simulink model "x_vacc_test"  (Simulink 8.0)
settings: Solver=ode45  StartTime=0.0  StopTime=20*(4096/8)*40  RelTol=1e-3
libraries: xbsIndex_r4 x531
(part 2 of 4)

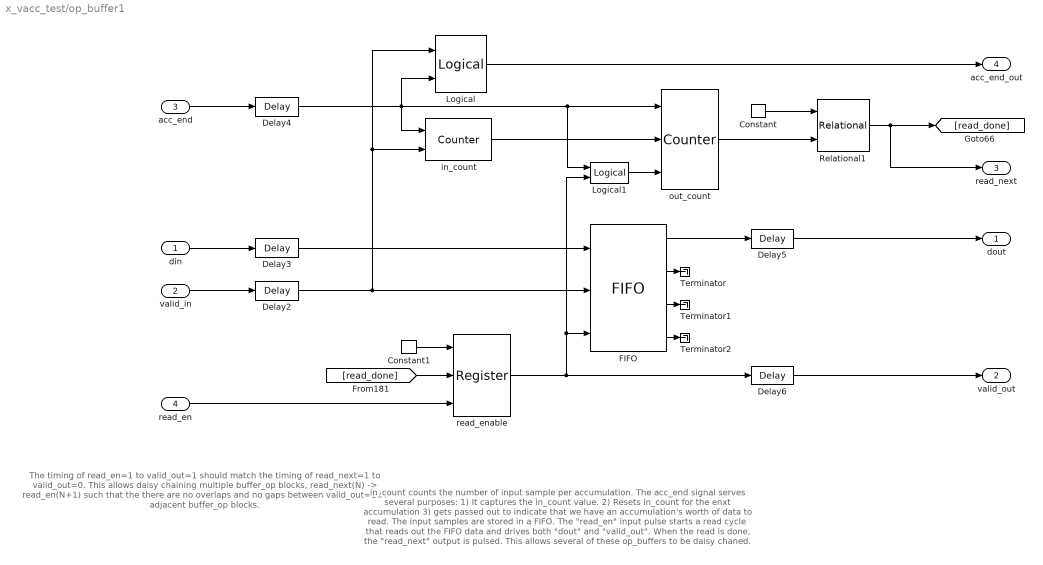
== system x_vacc_test/op_buffer1 ==
  note: The timing of read_en=1 to valid_out=1 should
      | match the timing of read_next=1 to valid_out=0.
      |
      | This allows daisy chaining multiple buffer_op blocks,
      | read_next(N) -> read_en(N+1) such that the there are
      | no overlaps and no gaps between valid_out=1 of
      | adjacent buffer_op blocks.
  note: in_count counts the number of input sample per accumulation.
      |
      | The acc_end signal serves several purposes:
      |
      | 1) It captures the in_count value.
      | 2) Resets in_count for the enxt accumulation
      | 3) gets passed out to indicate that we have an accumulation's
      | worth of data  to read.
      |
      | The input samples are stored in a FIFO.  The "read_en" input pulse
      | starts a read cycle that reads out the FIFO data and drives both
      | "dout" and "valid_out".  When the read is done, the "read_next"
      | output is pulsed.  This allows several of these op_buffers to be
      | daisy chaned.
  din : Inport
  valid_in : Inport  Port=2
  acc_end : Inport  Port=3
  read_en : Inport  Port=4
  Constant : xbsIndex_r4/Constant  const=1  gui_display_data_type=Fixed-point  arith_type=Unsigned  n_bits=1  bin_pt=0  preci_type=Single  exp_width=8  frac_width=24  explicit_period=on  period=1  equ=P=C  opselect=C  inp2=PCIN>>17  opr=+  inp1=P  carry=CIN  dbl_ovrd=off  block_type=constant
  Constant1 : xbsIndex_r4/Constant  const=1  gui_display_data_type=Boolean  arith_type=Boolean  n_bits=16  bin_pt=14  preci_type=Single  exp_width=8  frac_width=24  explicit_period=on  period=1  equ=P=C  opselect=C  inp2=PCIN>>17  opr=+  inp1=P  carry=CIN  dbl_ovrd=off  block_type=constant
  Delay{2..6} (5 alike) : xbsIndex_r4/Delay  rst=off  infoeditControl=Selection of Reset will increase slice count due to use of real FFs and instead of SRLs  en=off  latency=1  dbl_ovrd=off  reg_retiming=on  block_type=delay
      settings: block_version=11.4
  FIFO : xbsIndex_r4/FIFO  mem_type=Block RAM  performance_options=First_Word_Fall_Through  infoedit1=Available only for V4, V5, V6, V7, K7, A7 and Zynq devices.  embedded_registers=on  depth=8K  percent_nbits=1  rst=off  en=off  use_dcount=off  use_percent_full_port=on  use_almost_empty=off  almost_empty_thresh=2  use_almost_full=off  almost_full_thresh=14  dbl_ovrd=off  explicit_period=off  block_type=fifo
  Logical : xbsIndex_r4/Logical  logical_function=AND  inputs=2  en=off  latency=1  precision=Full  arith_type=Unsigned  n_bits=16  bin_pt=0  align_bp=on  dbl_ovrd=off  block_type=logical
  Logical1 : xbsIndex_r4/Logical  logical_function=OR  inputs=2  en=off  latency=0  precision=Full  arith_type=Unsigned  n_bits=16  bin_pt=0  align_bp=on  dbl_ovrd=off  block_type=logical
  Relational1 : xbsIndex_r4/Relational  mode=a=b  en=off  latency=1  dbl_ovrd=off  block_type=relational
  Terminator : Terminator
  Terminator1 : Terminator
  Terminator2 : Terminator
  in_count : xbsIndex_r4/Counter  cnt_type=Free Running  cnt_to=n_buffer * n_x_op * (2 ^ (freq_chan - n_x_eng)) - 1  operation=Up  start_count=0  cnt_by_val=1  arith_type=Unsigned  n_bits=nextpow2((n_x_op/2) * (2 ^ (freq_chan - n_x_eng)))  bin_pt=0  load_pin=off  rst=on  en=on  explicit_period=off  period=1  dbl_ovrd=off  use_behavioral_HDL=off  implementation=Fabric  block_type=counter
      settings: block_version=11.4
  out_count : xbsIndex_r4/Counter  cnt_type=Free Running  cnt_to=n_buffer * n_x_op * (2 ^ (freq_chan - n_x_eng)) - 1  operation=Down  start_count=0  cnt_by_val=1  arith_type=Unsigned  n_bits=nextpow2((n_x_op/2) * (2 ^ (freq_chan - n_x_eng)))  bin_pt=0  load_pin=on  rst=off  en=on  explicit_period=off  period=1  dbl_ovrd=off  use_behavioral_HDL=off  implementation=Fabric  block_type=counter
      settings: block_version=11.4
  read_enable : xbsIndex_r4/Register  init=0  rst=on  en=on  dbl_ovrd=off  block_type=register
  dout : Outport
  valid_out : Outport  Port=2
  read_next : Outport  Port=3
  acc_end_out : Outport  Port=4
  wires:
  acc_end/1 -> Delay4/1
  in_count/1 -> out_count/2
  valid_in/1 -> Delay2/1
  read_enable/1 -> Delay6/1, Logical1/2, FIFO/3
  out_count/1 -> Relational1/2
  din/1 -> Delay3/1
  Relational1/1 -> read_next/1, [read_done]
  [read_done] -> read_enable/2
  Delay6/1 -> valid_out/1
  FIFO/1 -> Delay5/1
  Logical/1 -> acc_end_out/1
  read_en/1 -> read_enable/3
  Delay2/1 -> FIFO/2, Logical/1, in_count/2
  Delay3/1 -> FIFO/1
  Delay4/1 -> Logical/2, out_count/1, Logical1/1, in_count/1
  FIFO/2 -> Terminator/1
  FIFO/3 -> Terminator1/1
  FIFO/4 -> Terminator2/1
  Constant/1 -> Relational1/1
  Constant1/1 -> read_enable/1
  Delay5/1 -> dout/1
  Logical1/1 -> out_count/3

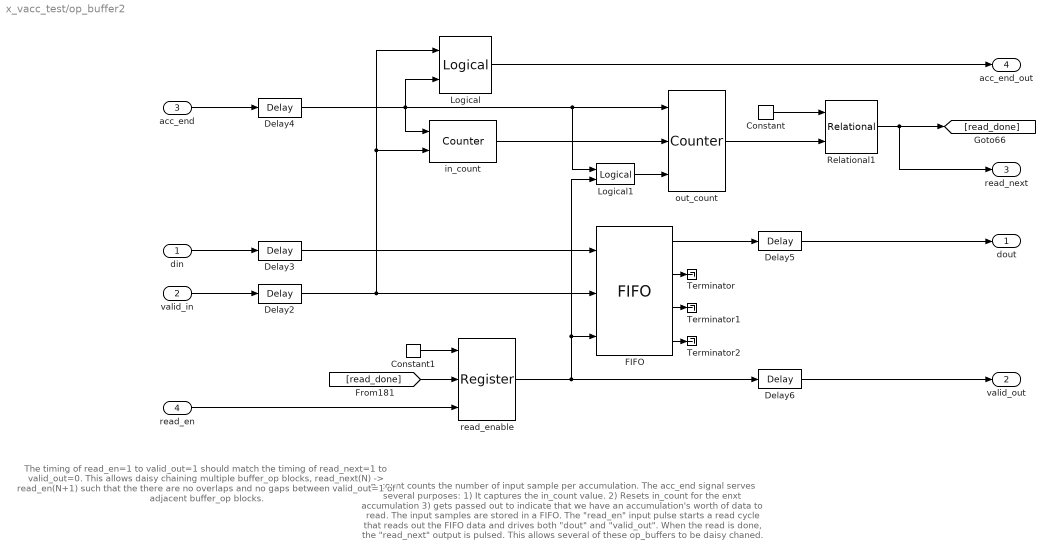
== system x_vacc_test/op_buffer2 ==
  note: in_count counts the number of input sample per accumulation.
      |
      | The acc_end signal serves several purposes:
      |
      | 1) It captures the in_count value.
      | 2) Resets in_count for the enxt accumulation
      | 3) gets passed out to indicate that we have an accumulation's
      | worth of data  to read.
      |
      | The input samples are stored in a FIFO.  The "read_en" input pulse
      | starts a read cycle that reads out the FIFO data and drives both
      | "dout" and "valid_out".  When the read is done, the "read_next"
      | output is pulsed.  This allows several of these op_buffers to be
      | daisy chaned.
  note: The timing of read_en=1 to valid_out=1 should
      | match the timing of read_next=1 to valid_out=0.
      |
      | This allows daisy chaining multiple buffer_op blocks,
      | read_next(N) -> read_en(N+1) such that the there are
      | no overlaps and no gaps between valid_out=1 of
      | adjacent buffer_op blocks.
  din : Inport
  valid_in : Inport  Port=2
  acc_end : Inport  Port=3
  read_en : Inport  Port=4
  Constant : xbsIndex_r4/Constant  const=1  gui_display_data_type=Fixed-point  arith_type=Unsigned  n_bits=1  bin_pt=0  preci_type=Single  exp_width=8  frac_width=24  explicit_period=on  period=1  equ=P=C  opselect=C  inp2=PCIN>>17  opr=+  inp1=P  carry=CIN  dbl_ovrd=off  block_type=constant
  Constant1 : xbsIndex_r4/Constant  const=1  gui_display_data_type=Boolean  arith_type=Boolean  n_bits=16  bin_pt=14  preci_type=Single  exp_width=8  frac_width=24  explicit_period=on  period=1  equ=P=C  opselect=C  inp2=PCIN>>17  opr=+  inp1=P  carry=CIN  dbl_ovrd=off  block_type=constant
  Delay{2..6} (5 alike) : xbsIndex_r4/Delay  rst=off  infoeditControl=Selection of Reset will increase slice count due to use of real FFs and instead of SRLs  en=off  latency=1  dbl_ovrd=off  reg_retiming=on  block_type=delay
      settings: block_version=11.4
  FIFO : xbsIndex_r4/FIFO  mem_type=Block RAM  performance_options=First_Word_Fall_Through  infoedit1=Available only for V4, V5, V6, V7, K7, A7 and Zynq devices.  embedded_registers=on  depth=8K  percent_nbits=1  rst=off  en=off  use_dcount=off  use_percent_full_port=on  use_almost_empty=off  almost_empty_thresh=2  use_almost_full=off  almost_full_thresh=14  dbl_ovrd=off  explicit_period=off  block_type=fifo
  Logical : xbsIndex_r4/Logical  logical_function=AND  inputs=2  en=off  latency=1  precision=Full  arith_type=Unsigned  n_bits=16  bin_pt=0  align_bp=on  dbl_ovrd=off  block_type=logical
  Logical1 : xbsIndex_r4/Logical  logical_function=OR  inputs=2  en=off  latency=0  precision=Full  arith_type=Unsigned  n_bits=16  bin_pt=0  align_bp=on  dbl_ovrd=off  block_type=logical
  Relational1 : xbsIndex_r4/Relational  mode=a=b  en=off  latency=1  dbl_ovrd=off  block_type=relational
  Terminator : Terminator
  Terminator1 : Terminator
  Terminator2 : Terminator
  in_count : xbsIndex_r4/Counter  cnt_type=Free Running  cnt_to=n_buffer * n_x_op * (2 ^ (freq_chan - n_x_eng)) - 1  operation=Up  start_count=0  cnt_by_val=1  arith_type=Unsigned  n_bits=nextpow2((n_x_op/2) * (2 ^ (freq_chan - n_x_eng)))  bin_pt=0  load_pin=off  rst=on  en=on  explicit_period=off  period=1  dbl_ovrd=off  use_behavioral_HDL=off  implementation=Fabric  block_type=counter
      settings: block_version=11.4
  out_count : xbsIndex_r4/Counter  cnt_type=Free Running  cnt_to=n_buffer * n_x_op * (2 ^ (freq_chan - n_x_eng)) - 1  operation=Down  start_count=0  cnt_by_val=1  arith_type=Unsigned  n_bits=nextpow2((n_x_op/2) * (2 ^ (freq_chan - n_x_eng)))  bin_pt=0  load_pin=on  rst=off  en=on  explicit_period=off  period=1  dbl_ovrd=off  use_behavioral_HDL=off  implementation=Fabric  block_type=counter
      settings: block_version=11.4
  read_enable : xbsIndex_r4/Register  init=0  rst=on  en=on  dbl_ovrd=off  block_type=register
  dout : Outport
  valid_out : Outport  Port=2
  read_next : Outport  Port=3
  acc_end_out : Outport  Port=4
  wires:
  Logical1/1 -> out_count/3
  Delay5/1 -> dout/1
  Constant1/1 -> read_enable/1
  Constant/1 -> Relational1/1
  FIFO/4 -> Terminator2/1
  FIFO/3 -> Terminator1/1
  FIFO/2 -> Terminator/1
  Delay4/1 -> in_count/1, Logical1/1, out_count/1, Logical/2
  Delay3/1 -> FIFO/1
  Delay2/1 -> in_count/2, Logical/1, FIFO/2
  read_en/1 -> read_enable/3
  Logical/1 -> acc_end_out/1
  FIFO/1 -> Delay5/1
  Delay6/1 -> valid_out/1
  [read_done] -> read_enable/2
  Relational1/1 -> [read_done], read_next/1
  din/1 -> Delay3/1
  out_count/1 -> Relational1/2
  read_enable/1 -> FIFO/3, Logical1/2, Delay6/1
  valid_in/1 -> Delay2/1
  in_count/1 -> out_count/2
  acc_end/1 -> Delay4/1

== system x_vacc_test/op_buffer3 ==
  note: The timing of read_en=1 to valid_out=1 should
      | match the timing of read_next=1 to valid_out=0.
      |
      | This allows daisy chaining multiple buffer_op blocks,
      | read_next(N) -> read_en(N+1) such that the there are
      | no overlaps and no gaps between valid_out=1 of
      | adjacent buffer_op blocks.
  note: in_count counts the number of input sample per accumulation.
      |
      | The acc_end signal serves several purposes:
      |
      | 1) It captures the in_count value.
      | 2) Resets in_count for the enxt accumulation
      | 3) gets passed out to indicate that we have an accumulation's
      | worth of data  to read.
      |
      | The input samples are stored in a FIFO.  The "read_en" input pulse
      | starts a read cycle that reads out the FIFO data and drives both
      | "dout" and "valid_out".  When the read is done, the "read_next"
      | output is pulsed.  This allows several of these op_buffers to be
      | daisy chaned.
  din : Inport
  valid_in : Inport  Port=2
  acc_end : Inport  Port=3
  read_en : Inport  Port=4
  Constant : xbsIndex_r4/Constant  const=1  gui_display_data_type=Fixed-point  arith_type=Unsigned  n_bits=1  bin_pt=0  preci_type=Single  exp_width=8  frac_width=24  explicit_period=on  period=1  equ=P=C  opselect=C  inp2=PCIN>>17  opr=+  inp1=P  carry=CIN  dbl_ovrd=off  block_type=constant
  Constant1 : xbsIndex_r4/Constant  const=1  gui_display_data_type=Boolean  arith_type=Boolean  n_bits=16  bin_pt=14  preci_type=Single  exp_width=8  frac_width=24  explicit_period=on  period=1  equ=P=C  opselect=C  inp2=PCIN>>17  opr=+  inp1=P  carry=CIN  dbl_ovrd=off  block_type=constant
  Delay{2..6} (5 alike) : xbsIndex_r4/Delay  rst=off  infoeditControl=Selection of Reset will increase slice count due to use of real FFs and instead of SRLs  en=off  latency=1  dbl_ovrd=off  reg_retiming=on  block_type=delay
      settings: block_version=11.4
  FIFO : xbsIndex_r4/FIFO  mem_type=Block RAM  performance_options=First_Word_Fall_Through  infoedit1=Available only for V4, V5, V6, V7, K7, A7 and Zynq devices.  embedded_registers=on  depth=8K  percent_nbits=1  rst=off  en=off  use_dcount=off  use_percent_full_port=on  use_almost_empty=off  almost_empty_thresh=2  use_almost_full=off  almost_full_thresh=14  dbl_ovrd=off  explicit_period=off  block_type=fifo
  Logical : xbsIndex_r4/Logical  logical_function=AND  inputs=2  en=off  latency=1  precision=Full  arith_type=Unsigned  n_bits=16  bin_pt=0  align_bp=on  dbl_ovrd=off  block_type=logical
  Logical1 : xbsIndex_r4/Logical  logical_function=OR  inputs=2  en=off  latency=0  precision=Full  arith_type=Unsigned  n_bits=16  bin_pt=0  align_bp=on  dbl_ovrd=off  block_type=logical
  Relational1 : xbsIndex_r4/Relational  mode=a=b  en=off  latency=1  dbl_ovrd=off  block_type=relational
  Terminator : Terminator
  Terminator1 : Terminator
  Terminator2 : Terminator
  in_count : xbsIndex_r4/Counter  cnt_type=Free Running  cnt_to=n_buffer * n_x_op * (2 ^ (freq_chan - n_x_eng)) - 1  operation=Up  start_count=0  cnt_by_val=1  arith_type=Unsigned  n_bits=nextpow2((n_x_op/2) * (2 ^ (freq_chan - n_x_eng)))  bin_pt=0  load_pin=off  rst=on  en=on  explicit_period=off  period=1  dbl_ovrd=off  use_behavioral_HDL=off  implementation=Fabric  block_type=counter
      settings: block_version=11.4
  out_count : xbsIndex_r4/Counter  cnt_type=Free Running  cnt_to=n_buffer * n_x_op * (2 ^ (freq_chan - n_x_eng)) - 1  operation=Down  start_count=0  cnt_by_val=1  arith_type=Unsigned  n_bits=nextpow2((n_x_op/2) * (2 ^ (freq_chan - n_x_eng)))  bin_pt=0  load_pin=on  rst=off  en=on  explicit_period=off  period=1  dbl_ovrd=off  use_behavioral_HDL=off  implementation=Fabric  block_type=counter
      settings: block_version=11.4
  read_enable : xbsIndex_r4/Register  init=0  rst=on  en=on  dbl_ovrd=off  block_type=register
  dout : Outport
  valid_out : Outport  Port=2
  read_next : Outport  Port=3
  acc_end_out : Outport  Port=4
  wires:
  acc_end/1 -> Delay4/1
  in_count/1 -> out_count/2
  valid_in/1 -> Delay2/1
  read_enable/1 -> Delay6/1, Logical1/2, FIFO/3
  out_count/1 -> Relational1/2
  din/1 -> Delay3/1
  Relational1/1 -> read_next/1, [read_done]
  [read_done] -> read_enable/2
  Delay6/1 -> valid_out/1
  FIFO/1 -> Delay5/1
  Logical/1 -> acc_end_out/1
  read_en/1 -> read_enable/3
  Delay2/1 -> FIFO/2, Logical/1, in_count/2
  Delay3/1 -> FIFO/1
  Delay4/1 -> Logical/2, out_count/1, Logical1/1, in_count/1
  FIFO/2 -> Terminator/1
  FIFO/3 -> Terminator1/1
  FIFO/4 -> Terminator2/1
  Constant/1 -> Relational1/1
  Constant1/1 -> read_enable/1
  Delay5/1 -> dout/1
  Logical1/1 -> out_count/3

== system x_vacc_test/op_buffer_to_workspace ==
  data : Inport
  valid : Inport  Port=2
  eof : Inport  Port=3
  BitBasher1 : xbsIndex_r4/BitBasher  bitexpr=|  display_expr=off  sr_1=1  arith_type1="Signed  (2's comp)"  bin_pt1=0  sr_2=2  arith_type2=Unsigned  bin_pt2=0  sr_3=3  arith_type3=Unsigned  bin_pt3=0  sr_4=4  arith_type4=Unsigned  bin_pt4=0  dbl_ovrd=off  block_type=bitbasher
      | <!DOCTYPE HTML PUBLIC "-//W3C//DTD HTML 4.0//EN" "http://www.w3.org/TR/REC-html40/strict.dtd">
      | <html><head><meta name="qrichtext" content="1" /><style type="text/css">
      | p, li { white-space: pre-wrap; }
      | </style></head><body style=" font-family:'Sans'; font-size:10pt; font-weight:400; font-style:normal;">
      | <p style=" margin-top:12px; margin-bottom:12px; margin-left:0px; margin-right:0px; -qt-block-indent:0; text-indent:0px;"><span style=" font-family:'MS Shell Dlg'; font-size:8pt;">d16={d[55:48],d[63:56]}</span></p></body></html>
  To Workspace : ToWorkspace  VariableName=xdata  SampleTime=-1
  To Workspace1 : ToWorkspace  VariableName=xvalid  SampleTime=-1
  To Workspace2 : ToWorkspace  VariableName=xeof  SampleTime=-1
  x_data : xbsIndex_r4/Gateway Out  inherit_from_input=off  hdl_port=off  timing_constraint=None  locs_specified=off  LOCs={}  UseAsDAC=off  DACChannel='1'  block_type=gatewayout
  x_eof : xbsIndex_r4/Gateway Out  inherit_from_input=off  hdl_port=off  timing_constraint=None  locs_specified=off  LOCs={}  UseAsDAC=off  DACChannel='1'  block_type=gatewayout
  x_valid : xbsIndex_r4/Gateway Out  inherit_from_input=off  hdl_port=off  timing_constraint=None  locs_specified=off  LOCs={}  UseAsDAC=off  DACChannel='1'  block_type=gatewayout
  wires:
  eof/1 -> x_eof/1
  x_valid/1 -> To Workspace1/1
  valid/1 -> x_valid/1
  BitBasher1/1 -> x_data/1  "dout"
  x_data/1 -> To Workspace/1
  data/1 -> BitBasher1/1
  x_eof/1 -> To Workspace2/1

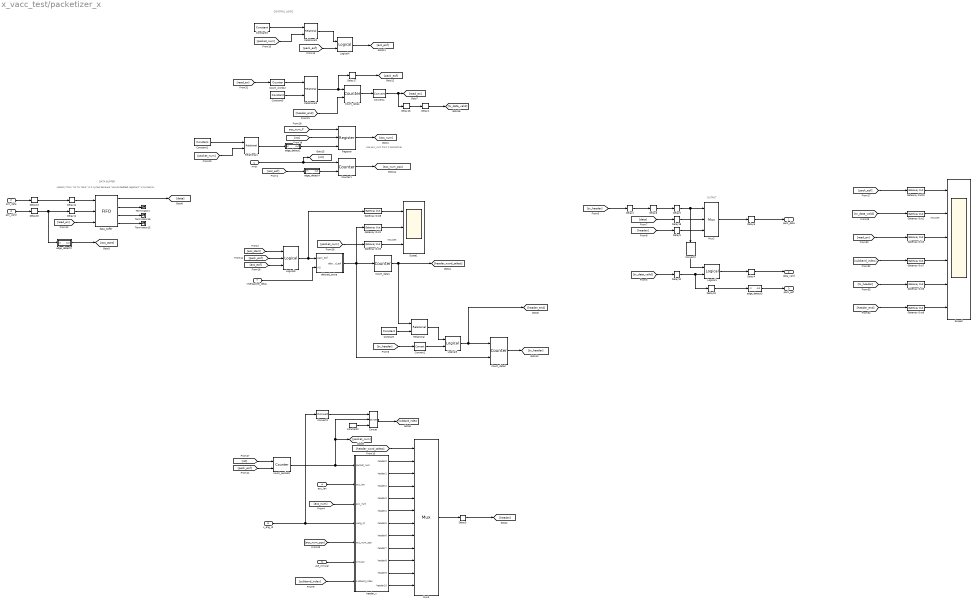
== system x_vacc_test/packetizer_x ==
  note: Use acc_num from P packetizer
  note: Latency from "re" to "dout" is 2 cycles
      | because "use embedded registers" is turned on.
  note: CONTROL LOGIC
  note: DATA BUFFER
  note: HEADER
  note: OUTPUT
  note: HEADER
  mrst : Inport
  acc_data : Inport  Port=2
  acc_valid : Inport  Port=3
  acc_len : Inport  Port=4
  use_circular : Inport  Port=5
  x_eng_id : Inport  Port=6
  interpacket_delay : Inport  Port=7
  Concat : xbsIndex_r4/Concat  num_inputs=3  dbl_ovrd=off  block_type=concat
  Constant : xbsIndex_r4/Constant  const=header_len / 8 - 3  gui_display_data_type=Fixed-point  arith_type=Unsigned  n_bits=ceil( log2( header_len / 8 ) )  bin_pt=0  preci_type=Single  exp_width=8  frac_width=24  explicit_period=on  period=1  equ=P=C  opselect=C  inp2=PCIN>>17  opr=+  inp1=P  carry=CIN  dbl_ovrd=off  block_type=constant
      settings: block_version=11.4
  Constant1 : xbsIndex_r4/Constant  const=0  gui_display_data_type=Fixed-point  arith_type=Unsigned  n_bits=ceil( log2( n_packets - 1 ) )  bin_pt=0  preci_type=Single  exp_width=8  frac_width=24  explicit_period=on  period=1  equ=P=C  opselect=C  inp2=PCIN>>17  opr=+  inp1=P  carry=CIN  dbl_ovrd=off  block_type=constant
      settings: block_version=11.4
  Constant2 : xbsIndex_r4/Constant  const=0  gui_display_data_type=Fixed-point  arith_type=Unsigned  n_bits=log2( n_freq_chan / n_packets )  bin_pt=0  preci_type=Single  exp_width=8  frac_width=24  explicit_period=on  period=1  equ=P=C  opselect=C  inp2=PCIN>>17  opr=+  inp1=P  carry=CIN  dbl_ovrd=off  block_type=constant
      settings: block_version=11.4
  Constant3 : xbsIndex_r4/Constant  const=n_packets - 1  gui_display_data_type=Fixed-point  arith_type=Unsigned  n_bits=ceil( log2( n_packets - 1 ) )  bin_pt=0  preci_type=Single  exp_width=8  frac_width=24  explicit_period=on  period=1  equ=P=C  opselect=C  inp2=PCIN>>17  opr=+  inp1=P  carry=CIN  dbl_ovrd=off  block_type=constant
      settings: block_version=11.4
  Constant5 : xbsIndex_r4/Constant  const=data_len / 8 - 2  gui_display_data_type=Fixed-point  arith_type=Unsigned  n_bits=ceil( log2( data_len / 8 ) )  bin_pt=0  preci_type=Single  exp_width=8  frac_width=24  explicit_period=on  period=1  equ=P=C  opselect=C  inp2=PCIN>>17  opr=+  inp1=P  carry=CIN  dbl_ovrd=off  block_type=constant
      settings: block_version=11.4
  Convert1 : xbsIndex_r4/Convert  gui_display_data_type=Fixed-point  arith_type=Boolean  n_bits=16  bin_pt=14  float_type=Single  exp_bits=8  fraction_bits=24  quantization=Truncate  overflow=Wrap  en=off  latency=1  dbl_ovrd=off  pipeline=off  block_type=convert
      settings: block_version=11.4
  Convert2 : xbsIndex_r4/Convert  gui_display_data_type=Fixed-point  arith_type=Boolean  n_bits=1  bin_pt=0  float_type=Single  exp_bits=8  fraction_bits=24  quantization=Truncate  overflow=Wrap  en=off  latency=0  dbl_ovrd=off  pipeline=on  block_type=convert
      settings: block_version=11.4
  Convert3 : xbsIndex_r4/Convert  gui_display_data_type=Fixed-point  arith_type=Unsigned  n_bits=n_x  bin_pt=0  float_type=Single  exp_bits=8  fraction_bits=24  quantization=Truncate  overflow=Wrap  en=off  latency=0  dbl_ovrd=off  pipeline=off  block_type=convert
      settings: block_version=11.4
  Convert4 : xbsIndex_r4/Convert  gui_display_data_type=Fixed-point  arith_type=Boolean  n_bits=16  bin_pt=14  float_type=Single  exp_bits=8  fraction_bits=24  quantization=Truncate  overflow=Wrap  en=off  latency=0  dbl_ovrd=off  pipeline=off  block_type=convert
      settings: block_version=11.4
  Count_data1 : xbsIndex_r4/Counter  cnt_type=Count Limited  cnt_to=header_len / 8 - 1  operation=Up  start_count=0  cnt_by_val=1  arith_type=Unsigned  n_bits=ceil( log2( header_len / 8 - 1 ) )  bin_pt=0  load_pin=off  rst=on  en=off  explicit_period=on  period=1  dbl_ovrd=off  use_behavioral_HDL=on  implementation=Fabric  use_rpm=off  block_type=counter
  Count_data2 : xbsIndex_r4/Counter  cnt_type=Free Running  cnt_to=1  operation=Up  start_count=0  cnt_by_val=1  arith_type=Unsigned  n_bits=1  bin_pt=0  load_pin=off  rst=on  en=on  explicit_period=off  period=1  dbl_ovrd=off  use_behavioral_HDL=on  implementation=Fabric  use_rpm=off  block_type=counter
  Count_data4 : xbsIndex_r4/Counter  cnt_type=Free Running  cnt_to=1  operation=Up  start_count=0  cnt_by_val=1  arith_type=Unsigned  n_bits=1  bin_pt=0  load_pin=off  rst=on  en=on  explicit_period=off  period=1  dbl_ovrd=off  use_behavioral_HDL=on  implementation=Fabric  use_rpm=off  block_type=counter
  Count_words2 : xbsIndex_r4/Counter  cnt_type=Count Limited  cnt_to=data_len / 8 - 1  operation=Up  start_count=0  cnt_by_val=1  arith_type=Unsigned  n_bits=ceil( log2( data_len / 8 ) )  bin_pt=0  load_pin=off  rst=off  en=on  explicit_period=off  period=1  dbl_ovrd=off  use_behavioral_HDL=on  implementation=Fabric  use_rpm=off  block_type=counter
  Counter1 : xbsIndex_r4/Counter  cnt_type=Count Limited  cnt_to=49  operation=Up  start_count=0  cnt_by_val=1  arith_type=Unsigned  n_bits=8  bin_pt=0  load_pin=off  rst=on  en=on  explicit_period=off  period=1  dbl_ovrd=off  use_behavioral_HDL=off  implementation=Fabric  block_type=counter
      settings: block_version=11.4
  Delay{1..6,8..11,13..19} (17 alike) : xbsIndex_r4/Delay  rst=off  infoeditControl=Selection of Reset will increase slice count due to use of real FFs and instead of SRLs  en=off  latency=1  dbl_ovrd=off  reg_retiming=on  block_type=delay
      settings: block_version=11.4
  Gateway Out{1..3,5..10} (9 alike) : xbsIndex_r4/Gateway Out  inherit_from_input=off  hdl_port=off  timing_constraint=None  locs_specified=off  LOCs={}  UseAsDAC=off  DACChannel='1'  block_type=gatewayout
      settings: infoedit="Gateway out block.  Converts Xilinx fixed point inputs into ouputs of type Simulink integer, double, or fixed point.<br><br>Hardware notes:  In hardware these blocks become top level output ports or are discarded, depending on how they are configured."  block_version=11.4
  Logical1 : xbsIndex_r4/Logical  logical_function=OR  inputs=2  en=off  latency=1  precision=Full  arith_type=Unsigned  n_bits=16  bin_pt=0  align_bp=on  dbl_ovrd=off  block_type=logical
      settings: block_version=11.4
  Logical3 : xbsIndex_r4/Logical  logical_function=AND  inputs=2  en=off  latency=1  precision=Full  arith_type=Unsigned  n_bits=16  bin_pt=0  align_bp=on  dbl_ovrd=off  block_type=logical
      settings: block_version=11.4
  Logical4 : xbsIndex_r4/Logical  logical_function=AND  inputs=2  en=off  latency=0  precision=Full  arith_type=Unsigned  n_bits=16  bin_pt=0  align_bp=on  dbl_ovrd=off  block_type=logical
      settings: block_version=11.4
  Logical9 : xbsIndex_r4/Logical  logical_function=XOR  inputs=3  en=off  latency=0  precision=Full  arith_type=Unsigned  n_bits=16  bin_pt=0  align_bp=on  dbl_ovrd=off  block_type=logical
      settings: block_version=11.4
  Mux2 : xbsIndex_r4/Mux  inputs=2  en=off  latency=1  precision=Full  arith_type=Unsigned  n_bits=16  bin_pt=14  quantization=Truncate  overflow=Wrap  dbl_ovrd=off  block_type=mux
      settings: block_version=11.4
  Mux3 : xbsIndex_r4/Mux  inputs=11  en=off  latency=1  precision=Full  arith_type=Unsigned  n_bits=16  bin_pt=14  quantization=Truncate  overflow=Wrap  dbl_ovrd=off  block_type=mux
      settings: block_version=11.4
  Register : xbsIndex_r4/Register  init=0  rst=on  en=on  dbl_ovrd=off  block_type=register
  Relational{,1..3} (4 alike) : xbsIndex_r4/Relational  mode=a=b  en=off  latency=1  dbl_ovrd=off  block_type=relational
  Scope1 : Scope  NumInputPorts=3
      settings: YMin=-5~-5~-5  YMax=5~5~5
  Scope2 : Scope  NumInputPorts=6
      settings: YMin=-5~-5~-5~-5~-5~-5  YMax=5~5~5~5~5~5  SaveName=ScopeData2
  Terminator1 : Terminator
  Terminator15 : Terminator
  Terminator16 : Terminator
  count_packets : xbsIndex_r4/Counter  cnt_type=Count Limited  cnt_to=n_packets - 1  operation=Up  start_count=0  cnt_by_val=1  arith_type=Unsigned  n_bits=ceil( log2( n_packets ) )  bin_pt=0  load_pin=off  rst=on  en=on  explicit_period=off  period=1  dbl_ovrd=off  use_behavioral_HDL=on  implementation=Fabric  use_rpm=off  block_type=counter
  data_buffer : xbsIndex_r4/FIFO  mem_type=Block RAM  performance_options=Standard_FIFO  infoedit1=Available only for V4, V5, V6, V7, K7, A7 and Zynq devices.  embedded_registers=on  depth=32K  percent_nbits=1  rst=off  en=off  use_dcount=off  use_percent_full_port=on  use_almost_empty=off  almost_empty_thresh=2  use_almost_full=off  almost_full_thresh=14  dbl_ovrd=off  explicit_period=off  block_type=fifo
      settings: block_version=10.1.3
  delayed_pulse : Subsystem
  edge_detect1 : Subsystem  AncestorBlock=casper_library_misc/edge_detect  mask(edge_detect){edge=Rising, polarity=Active High, x_in=[0 0.08 0.08 0.16 0.16 0.24 0.24 0.32 0.32 0.4], y_in=[1  1 -1 -1 -1 -1 -1 -1 -1 -1], x_out=[0.6 0.68 0.68 0.76 0.76 0.84 0.84 0.92 0.92 1], y_out=[-1 -1  1  1 -1 -1 -1 -1 -1 -1]}
      mask prompts: edge "Edge Type"; polarity "Output Polarity"; x_in "Input X Positions"; y_in "Input Y Positions"; x_out "Output X Positions"; y_out "Output Y Positions"
      mask help: Output is active for one-cycle after each edge of the specified type (i.e. rising, falling or both).
      mask init: same as x_vacc_test/edge_detect17
      settings: AttributesFormatString=|
      | rising edges
      | active high
      mask Help: Output is active for one-cycle after each edge of the specified type (i.e. rising, falling, or both).  Latency is zero.
      mask TypeOptions edge: Rising | Falling | Both
      mask TypeOptions polarity: Active High | Active Low
      mask Display:
      | plot(x_in_num,0.5+y_in_num*0.2);
      | plot(x_out_num,0.5+y_out_num*0.2);
  edge_detect2 : Subsystem  AncestorBlock=casper_library_misc/edge_detect  mask(edge_detect){edge=Rising, polarity=Active High, x_in=[0 0.08 0.08 0.16 0.16 0.24 0.24 0.32 0.32 0.4], y_in=[-1 -1  1  1  1  1  1  1  1  1], x_out=[0.6 0.68 0.68 0.76 0.76 0.84 0.84 0.92 0.92 1], y_out=[-1 -1  1  1 -1 -1 -1 -1 -1 -1]}
      mask prompts: edge "Edge Type"; polarity "Output Polarity"; x_in "Input X Positions"; y_in "Input Y Positions"; x_out "Output X Positions"; y_out "Output Y Positions"
      mask help: Output is active for one-cycle after each edge of the specified type (i.e. rising, falling or both).
      mask init: same as x_vacc_test/edge_detect17
      settings: AttributesFormatString=|
      | rising edges
      | active high
      mask Help: Output is active for one-cycle after each edge of the specified type (i.e. rising, falling, or both).  Latency is zero.
      mask TypeOptions edge: Rising | Falling | Both
      mask TypeOptions polarity: Active High | Active Low
      mask Display:
      | plot(x_in_num,0.5+y_in_num*0.2);
      | plot(x_out_num,0.5+y_out_num*0.2);
  edge_detect3 : Subsystem  AncestorBlock=casper_library_misc/edge_detect  mask(edge_detect){edge=Falling, polarity=Active High, x_in=[0 0.08 0.08 0.16 0.16 0.24 0.24 0.32 0.32 0.4], y_in=[1  1 -1 -1 -1 -1 -1 -1 -1 -1], x_out=[0.6 0.68 0.68 0.76 0.76 0.84 0.84 0.92 0.92 1], y_out=[-1 -1  1  1 -1 -1 -1 -1 -1 -1]}
      mask prompts: edge "Edge Type"; polarity "Output Polarity"; x_in "Input X Positions"; y_in "Input Y Positions"; x_out "Output X Positions"; y_out "Output Y Positions"
      mask help: Output is active for one-cycle after each edge of the specified type (i.e. rising, falling or both).
      mask init: same as x_vacc_test/edge_detect17
      settings: AttributesFormatString=|
      | falling edges
      | active high
      mask Help: Output is active for one-cycle after each edge of the specified type (i.e. rising, falling, or both).  Latency is zero.
      mask TypeOptions edge: Rising | Falling | Both
      mask TypeOptions polarity: Active High | Active Low
      mask Display:
      | plot(x_in_num,0.5+y_in_num*0.2);
      | plot(x_out_num,0.5+y_out_num*0.2);
  edge_detect4 : Subsystem  AncestorBlock=casper_library_misc/edge_detect  mask(edge_detect){edge=Falling, polarity=Active High, x_in=[0 0.08 0.08 0.16 0.16 0.24 0.24 0.32 0.32 0.4], y_in=[1  1 -1 -1 -1 -1 -1 -1 -1 -1], x_out=[0.6 0.68 0.68 0.76 0.76 0.84 0.84 0.92 0.92 1], y_out=[-1 -1  1  1 -1 -1 -1 -1 -1 -1]}
      mask prompts: edge "Edge Type"; polarity "Output Polarity"; x_in "Input X Positions"; y_in "Input Y Positions"; x_out "Output X Positions"; y_out "Output Y Positions"
      mask help: Output is active for one-cycle after each edge of the specified type (i.e. rising, falling or both).
      mask init: same as x_vacc_test/edge_detect17
      settings: AttributesFormatString=|
      | falling edges
      | active high
      mask Help: Output is active for one-cycle after each edge of the specified type (i.e. rising, falling, or both).  Latency is zero.
      mask TypeOptions edge: Rising | Falling | Both
      mask TypeOptions polarity: Active High | Active Low
      mask Display:
      | plot(x_in_num,0.5+y_in_num*0.2);
      | plot(x_out_num,0.5+y_out_num*0.2);
  header_x : Subsystem
  pack_data : Outport
  data_valid : Outport  Port=2
  pack_eof : Outport  Port=3
  wires:
  data_buffer/3 -> Terminator16/1
  data_buffer/4 -> Terminator15/1
  Delay14/1 -> data_buffer/1
  Delay11/1 -> data_buffer/2
  acc_data/1 -> Delay13/1
  acc_valid/1 -> Delay15/1
  Mux2/1 -> Delay6/1
  [tx_header] -> Delay1/1
  [read_en] -> data_buffer/3
  data_buffer/2 -> Terminator1/1
  [pack_eof] -> Logical9/2
  [acc_start] -> Logical9/1
  Count_data1/1 -> [header_word_select], Relational/1
  Constant/1 -> Relational/2
  Logical1/1 -> Delay4/1
  Delay1/1 -> Delay8/1
  Convert2/1 -> Logical1/1
  Logical3/1 -> [header_end], Count_data2/1
  Count_data2/1 -> [tx_header]
  Relational/1 -> Logical3/1
  Mux3/1 -> Delay5/1
  [data] -> Delay18/1
  [header] -> Delay9/1
  edge_detect2/1(out) -> [acc_start]
  [header_word_select] -> Mux3/1
  count_packets/1 -> header_x/1(packet_num), [packet_num], Concat/2
  acc_len/1 -> header_x/2(acc_len)
  [acc_num] -> header_x/3(acc_num)
  [acc_num_pps] -> header_x/5(acc_num_pps)
  use_circular/1 -> header_x/6(circular)
  Constant3/1 -> Relational2/1
  [packet_num] -> Relational2/2
  Relational2/1 -> Logical4/1
  Logical4/1 -> [acc_eof]
  [acc_eof] -> Logical9/3
  header_x/1(header0) -> Mux3/2
  header_x/2(header1) -> Mux3/3
  header_x/3(header2) -> Mux3/4
  header_x/4(header3) -> Mux3/5
  header_x/5(header4) -> Mux3/6
  header_x/6(header5) -> Mux3/7
  header_x/7(header6) -> Mux3/8
  header_x/8(header7) -> Mux3/9
  header_x/9(header8) -> Mux3/10
  header_x/10(header9) -> Mux3/11
  header_x/11(header10) -> Mux3/12
  x_eng_id/1 -> header_x/4(xeng_id), Convert3/1
  Concat/1 -> [subband_index]
  [subband_index] -> header_x/7(subband_index)
  [pack_eof] -> Logical4/2
  Delay3/1 -> Mux2/1, Convert2/1
  Delay5/1 -> [header]
  edge_detect3/1(out) -> pack_eof/1
  Delay4/1 -> data_valid/1
  Delay6/1 -> pack_data/1
  interpacket_delay/1 -> delayed_pulse/2(In2)
  delayed_pulse/1(delayed_eof) -> Count_data1/1, Gateway Out1/1, Count_data2/2
  Logical9/1 -> delayed_pulse/1(pack_eof), Gateway Out8/1
  Gateway Out8/1 -> Scope1/1
  Gateway Out1/1 -> Scope1/2
  Gateway Out5/1 -> Scope1/3
  mrst/1 -> Counter1/1, [rst]
  edge_detect4/1(out) -> Counter1/2
  Register/1 -> [acc_num]
  Counter1/1 -> [acc_num_pps]
  [acc_eof] -> edge_detect4/1(in)
  [packet_num] -> Gateway Out5/1
  [pack_eof] -> count_packets/2
  [rst] -> count_packets/1
  Delay8/1 -> Delay3/1
  Delay9/1 -> Mux2/3
  Gateway Out2/1 -> Scope2/2
  [tx_data_valid] -> Gateway Out2/1
  [read_en] -> Gateway Out3/1
  Gateway Out3/1 -> Scope2/3
  Count_words2/1 -> Relational3/1
  Constant5/1 -> Relational3/2
  Gateway Out7/1 -> Scope2/4
  [subband_index] -> Gateway Out7/1
  [tx_header] -> Gateway Out6/1
  Gateway Out6/1 -> Scope2/5
  [header_end] -> Gateway Out9/1
  Gateway Out9/1 -> Scope2/6
  [read_en] -> Count_words2/1
  Delay13/1 -> Delay14/1
  Delay15/1 -> Delay11/1, edge_detect2/1(in)
  [tx_header] -> Convert1/1
  Convert1/1 -> Logical3/2
  Count_data4/1 -> Convert4/1
  Relational3/1 -> Count_data4/1, Delay17/1
  [header_end] -> Count_data4/2
  Convert4/1 -> [read_en], Delay16/1
  data_buffer/1 -> [data]
  Gateway Out10/1 -> Scope2/1
  [pack_eof] -> Gateway Out10/1
  Delay17/1 -> [pack_eof]
  Constant2/1 -> Concat/3
  Convert3/1 -> Concat/1
  [tx_data_valid] -> Delay10/1
  Delay19/1 -> edge_detect3/1(in)
  Delay16/1 -> Delay2/1
  Delay18/1 -> Mux2/2
  Delay2/1 -> [tx_data_valid]
  Delay10/1 -> Logical1/2, Delay19/1
  [rst] -> Register/2
  [acc_num_P] -> Register/1
  Constant1/1 -> Relational1/1
  [packet_num] -> Relational1/2
  Relational1/1 -> edge_detect1/1(in)
  edge_detect1/1(out) -> Register/3

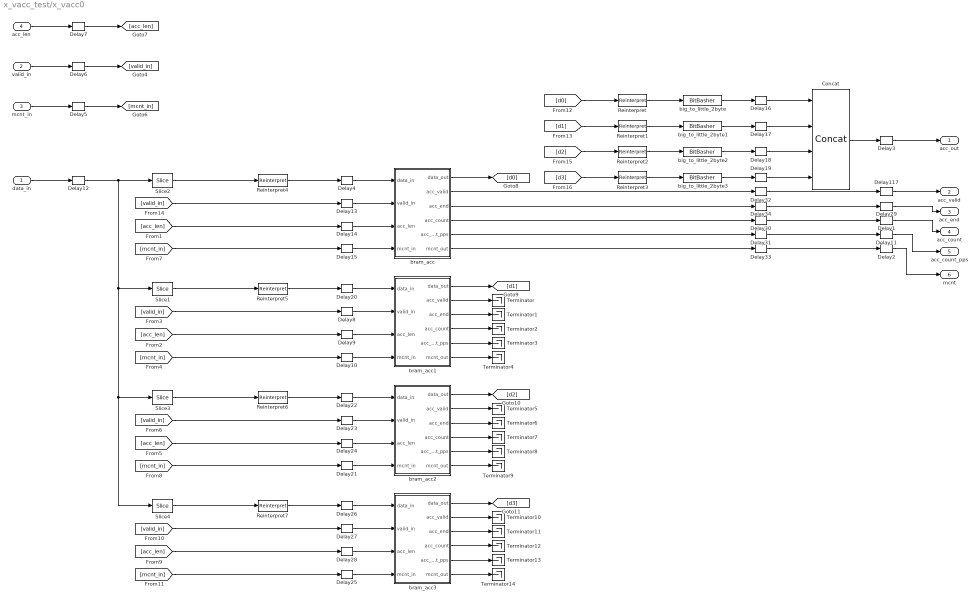
== system x_vacc_test/x_vacc0 ==
  data_in : Inport
  valid_in : Inport  Port=2
  mcnt_in : Inport  Port=3
  acc_len : Inport  Port=4
  Concat : xbsIndex_r4/Concat  num_inputs=4  dbl_ovrd=off  block_type=concat
  Delay{1..34,117} (35 alike) : xbsIndex_r4/Delay  rst=off  infoeditControl=Selection of Reset will increase slice count due to use of real FFs and instead of SRLs  en=off  latency=1  dbl_ovrd=off  reg_retiming=on  block_type=delay
      settings: block_version=11.4
  Reinterpret{,1..3} (4 alike) : xbsIndex_r4/Reinterpret  force_arith_type=on  arith_type=Unsigned  force_bin_pt=on  bin_pt=0  block_type=reinterpret
      settings: infoedit="Changes signal type without altering the binary representation.   You can changed the signal between signed and unsigned, and relocate the binary point.<br><br>Hardware notes: In hardware this block costs nothing.<br><br>Example:  Suppose the input is 6 bits wide, signed, with 2 fractional bits, and the output is forced to unsigned with 0 fractional bits.  Then an input of -2.0 (1110.00 in binary 2's complement) becomes an output of 56 (111000 in binary)."
  Reinterpret{4..7} (4 alike) : xbsIndex_r4/Reinterpret  force_arith_type=on  arith_type="Signed  (2's comp)"  force_bin_pt=on  bin_pt=x_bin_pt  block_type=reinterpret
      settings: infoedit="Changes signal type without altering the binary representation.   You can changed the signal between signed and unsigned, and relocate the binary point.<br><br>Hardware notes: In hardware this block costs nothing.<br><br>Example:  Suppose the input is 6 bits wide, signed, with 2 fractional bits, and the output is forced to unsigned with 0 fractional bits.  Then an input of -2.0 (1110.00 in binary 2's complement) becomes an output of 56 (111000 in binary)."
  Slice1 : xbsIndex_r4/Slice  nbits=16  boolean_output=off  mode=Lower Bit Location + Width  bit1=0  base1=MSB of Input  bit0=32  base0=LSB of Input  dbl_ovrd=off  block_type=slice
  Slice2 : xbsIndex_r4/Slice  nbits=16  boolean_output=off  mode=Upper Bit Location + Width  bit1=0  base1=MSB of Input  bit0=0  base0=LSB of Input  dbl_ovrd=off  block_type=slice
  Slice3 : xbsIndex_r4/Slice  nbits=16  boolean_output=off  mode=Lower Bit Location + Width  bit1=0  base1=MSB of Input  bit0=16  base0=LSB of Input  dbl_ovrd=off  block_type=slice
  Slice4 : xbsIndex_r4/Slice  nbits=16  boolean_output=off  mode=Lower Bit Location + Width  bit1=0  base1=MSB of Input  bit0=0  base0=LSB of Input  dbl_ovrd=off  block_type=slice
  Terminator{,1..14} (15 alike) : Terminator
  big_to_little_2byte : xbsIndex_r4/BitBasher  bitexpr=|  display_expr=off  sr_1=1  arith_type1=Unsigned  bin_pt1=0  sr_2=2  arith_type2=Unsigned  bin_pt2=0  sr_3=3  arith_type3=Unsigned  bin_pt3=0  sr_4=4  arith_type4=Unsigned  bin_pt4=0  dbl_ovrd=off  block_type=bitbasher
      | <!DOCTYPE HTML PUBLIC "-//W3C//DTD HTML 4.0//EN" "http://www.w3.org/TR/REC-html40/strict.dtd">
      | <html><head><meta name="qrichtext" content="1" /><style type="text/css">
      | p, li { white-space: pre-wrap; }
      | </style></head><body style=" font-family:'Sans Serif'; font-size:9pt; font-weight:400; font-style:normal;">
      | <p style=" margin-top:12px; margin-bottom:12px; margin-left:0px; margin-right:0px; -qt-block-indent:0; text-indent:0px; font-family:'MS Shell Dlg'; font-size:8pt;">y={x[7:0], x[15:8]}</p></body></html>
      settings: block_version=11.4
  big_to_little_2byte1 : xbsIndex_r4/BitBasher  bitexpr=|  display_expr=off  sr_1=1  arith_type1=Unsigned  bin_pt1=0  sr_2=2  arith_type2=Unsigned  bin_pt2=0  sr_3=3  arith_type3=Unsigned  bin_pt3=0  sr_4=4  arith_type4=Unsigned  bin_pt4=0  dbl_ovrd=off  block_type=bitbasher
      | <!DOCTYPE HTML PUBLIC "-//W3C//DTD HTML 4.0//EN" "http://www.w3.org/TR/REC-html40/strict.dtd">
      | <html><head><meta name="qrichtext" content="1" /><style type="text/css">
      | p, li { white-space: pre-wrap; }
      | </style></head><body style=" font-family:'Sans Serif'; font-size:9pt; font-weight:400; font-style:normal;">
      | <p style=" margin-top:12px; margin-bottom:12px; margin-left:0px; margin-right:0px; -qt-block-indent:0; text-indent:0px; font-family:'MS Shell Dlg'; font-size:8pt;">y={x[7:0], x[15:8]}</p></body></html>
      settings: block_version=11.4
  big_to_little_2byte2 : xbsIndex_r4/BitBasher  bitexpr=|  display_expr=off  sr_1=1  arith_type1=Unsigned  bin_pt1=0  sr_2=2  arith_type2=Unsigned  bin_pt2=0  sr_3=3  arith_type3=Unsigned  bin_pt3=0  sr_4=4  arith_type4=Unsigned  bin_pt4=0  dbl_ovrd=off  block_type=bitbasher
      | <!DOCTYPE HTML PUBLIC "-//W3C//DTD HTML 4.0//EN" "http://www.w3.org/TR/REC-html40/strict.dtd">
      | <html><head><meta name="qrichtext" content="1" /><style type="text/css">
      | p, li { white-space: pre-wrap; }
      | </style></head><body style=" font-family:'Sans Serif'; font-size:9pt; font-weight:400; font-style:normal;">
      | <p style=" margin-top:12px; margin-bottom:12px; margin-left:0px; margin-right:0px; -qt-block-indent:0; text-indent:0px; font-family:'MS Shell Dlg'; font-size:8pt;">y={x[7:0], x[15:8]}</p></body></html>
      settings: block_version=11.4
  big_to_little_2byte3 : xbsIndex_r4/BitBasher  bitexpr=|  display_expr=off  sr_1=1  arith_type1=Unsigned  bin_pt1=0  sr_2=2  arith_type2=Unsigned  bin_pt2=0  sr_3=3  arith_type3=Unsigned  bin_pt3=0  sr_4=4  arith_type4=Unsigned  bin_pt4=0  dbl_ovrd=off  block_type=bitbasher
      | <!DOCTYPE HTML PUBLIC "-//W3C//DTD HTML 4.0//EN" "http://www.w3.org/TR/REC-html40/strict.dtd">
      | <html><head><meta name="qrichtext" content="1" /><style type="text/css">
      | p, li { white-space: pre-wrap; }
      | </style></head><body style=" font-family:'Sans Serif'; font-size:9pt; font-weight:400; font-style:normal;">
      | <p style=" margin-top:12px; margin-bottom:12px; margin-left:0px; margin-right:0px; -qt-block-indent:0; text-indent:0px; font-family:'MS Shell Dlg'; font-size:8pt;">y={x[7:0], x[15:8]}</p></body></html>
      settings: block_version=11.4
  bram_acc : Subsystem
  bram_acc1 : Subsystem
  bram_acc2 : Subsystem
  bram_acc3 : Subsystem
  acc_out : Outport
  acc_valid : Outport  Port=2
  acc_end : Outport  Port=3
  acc_count : Outport  Port=4
  acc_count_pps : Outport  Port=5
  mcnt : Outport  Port=6
  wires:
  big_to_little_2byte3/1 -> Delay19/1
  big_to_little_2byte2/1 -> Delay18/1
  big_to_little_2byte1/1 -> Delay17/1
  big_to_little_2byte/1 -> Delay16/1
  Delay4/1 -> bram_acc/1(data_in)
  Delay3/1 -> acc_out/1
  Slice2/1 -> Reinterpret4/1
  [valid_in] -> Delay13/1
  Concat/1 -> Delay3/1
  data_in/1 -> Delay12/1
  mcnt_in/1 -> Delay5/1
  valid_in/1 -> Delay6/1
  acc_len/1 -> Delay7/1
  Delay6/1 -> [valid_in]
  Delay5/1 -> [mcnt_in]
  Delay7/1 -> [acc_len]
  Delay12/1 -> Slice2/1, Slice1/1, Slice4/1, Slice3/1
  [acc_len] -> Delay14/1
  [mcnt_in] -> Delay15/1
  Delay13/1 -> bram_acc/2(valid_in)
  Delay14/1 -> bram_acc/3(acc_len)
  Delay15/1 -> bram_acc/4(mcnt_in)
  Reinterpret/1 -> big_to_little_2byte/1
  Delay16/1 -> Concat/1
  Delay17/1 -> Concat/2
  Delay18/1 -> Concat/3
  Delay19/1 -> Concat/4
  Delay20/1 -> bram_acc1/1(data_in)
  Slice1/1 -> Reinterpret5/1
  [valid_in] -> Delay8/1
  [acc_len] -> Delay9/1
  [mcnt_in] -> Delay10/1
  Delay8/1 -> bram_acc1/2(valid_in)
  Delay9/1 -> bram_acc1/3(acc_len)
  Delay10/1 -> bram_acc1/4(mcnt_in)
  Delay22/1 -> bram_acc2/1(data_in)
  Slice3/1 -> Reinterpret6/1
  [valid_in] -> Delay23/1
  [acc_len] -> Delay24/1
  [mcnt_in] -> Delay21/1
  Delay23/1 -> bram_acc2/2(valid_in)
  Delay24/1 -> bram_acc2/3(acc_len)
  Delay21/1 -> bram_acc2/4(mcnt_in)
  Delay26/1 -> bram_acc3/1(data_in)
  Slice4/1 -> Reinterpret7/1
  [valid_in] -> Delay27/1
  [acc_len] -> Delay28/1
  [mcnt_in] -> Delay25/1
  Delay27/1 -> bram_acc3/2(valid_in)
  Delay28/1 -> bram_acc3/3(acc_len)
  Delay25/1 -> bram_acc3/4(mcnt_in)
  Reinterpret3/1 -> big_to_little_2byte3/1
  Reinterpret2/1 -> big_to_little_2byte2/1
  Reinterpret1/1 -> big_to_little_2byte1/1
  bram_acc/1(data_out) -> [d0]
  bram_acc2/1(data_out) -> [d2]
  bram_acc3/1(data_out) -> [d3]
  [d0] -> Reinterpret/1
  [d3] -> Reinterpret3/1
  [d2] -> Reinterpret2/1
  [d1] -> Reinterpret1/1
  Delay32/1 -> Delay117/1
  Delay34/1 -> Delay29/1
  Delay30/1 -> Delay1/1
  Delay31/1 -> Delay11/1
  Delay33/1 -> Delay2/1
  bram_acc/2(acc_valid) -> Delay32/1
  bram_acc/3(acc_end) -> Delay34/1
  bram_acc/4(acc_count) -> Delay30/1
  bram_acc/5(acc_count_pps) -> Delay31/1
  bram_acc/6(mcnt_out) -> Delay33/1
  Delay117/1 -> acc_valid/1
  Delay29/1 -> acc_end/1
  Delay1/1 -> acc_count/1
  Delay11/1 -> acc_count_pps/1
  Delay2/1 -> mcnt/1
  bram_acc2/2(acc_valid) -> Terminator5/1
  bram_acc2/3(acc_end) -> Terminator6/1
  bram_acc2/4(acc_count) -> Terminator7/1
  bram_acc2/5(acc_count_pps) -> Terminator8/1
  bram_acc2/6(mcnt_out) -> Terminator9/1
  bram_acc3/2(acc_valid) -> Terminator10/1
  bram_acc3/3(acc_end) -> Terminator11/1
  bram_acc3/4(acc_count) -> Terminator12/1
  bram_acc3/5(acc_count_pps) -> Terminator13/1
  bram_acc3/6(mcnt_out) -> Terminator14/1
  Reinterpret4/1 -> Delay4/1
  Reinterpret5/1 -> Delay20/1
  Reinterpret6/1 -> Delay22/1
  Reinterpret7/1 -> Delay26/1
  bram_acc1/1(data_out) -> [d1]
  bram_acc1/2(acc_valid) -> Terminator/1
  bram_acc1/3(acc_end) -> Terminator1/1
  bram_acc1/4(acc_count) -> Terminator2/1
  bram_acc1/5(acc_count_pps) -> Terminator3/1
  bram_acc1/6(mcnt_out) -> Terminator4/1

== system x_vacc_test/x_vacc_to_workspace ==
  dout : Inport
  vout : Inport  Port=2
  acc_end : Inport  Port=3
  acc_count : Inport  Port=4
  acc_count_pps : Inport  Port=5
  BitBasher1 : xbsIndex_r4/BitBasher  bitexpr=|  display_expr=off  sr_1=1  arith_type1="Signed  (2's comp)"  bin_pt1=0  sr_2=2  arith_type2=Unsigned  bin_pt2=0  sr_3=3  arith_type3=Unsigned  bin_pt3=0  sr_4=4  arith_type4=Unsigned  bin_pt4=0  dbl_ovrd=off  block_type=bitbasher
      | <!DOCTYPE HTML PUBLIC "-//W3C//DTD HTML 4.0//EN" "http://www.w3.org/TR/REC-html40/strict.dtd">
      | <html><head><meta name="qrichtext" content="1" /><style type="text/css">
      | p, li { white-space: pre-wrap; }
      | </style></head><body style=" font-family:'Sans'; font-size:10pt; font-weight:400; font-style:normal;">
      | <p style=" margin-top:12px; margin-bottom:12px; margin-left:0px; margin-right:0px; -qt-block-indent:0; text-indent:0px;"><span style=" font-family:'MS Shell Dlg'; font-size:8pt;">d16={d[55:48],d[63:56]}</span></p></body></html>
  To Workspace : ToWorkspace  VariableName=dout  SampleTime=-1
  To Workspace1 : ToWorkspace  VariableName=vout  SampleTime=-1
  To Workspace2 : ToWorkspace  VariableName=acc_end  SampleTime=-1
  To Workspace3 : ToWorkspace  VariableName=acc_count  SampleTime=-1
  To Workspace4 : ToWorkspace  VariableName=acc_count_pps  SampleTime=-1
  gw_acc_count : xbsIndex_r4/Gateway Out  inherit_from_input=off  hdl_port=off  timing_constraint=None  locs_specified=off  LOCs={}  UseAsDAC=off  DACChannel='1'  block_type=gatewayout
  gw_acc_count_pps : xbsIndex_r4/Gateway Out  inherit_from_input=off  hdl_port=off  timing_constraint=None  locs_specified=off  LOCs={}  UseAsDAC=off  DACChannel='1'  block_type=gatewayout
  gw_acc_end : xbsIndex_r4/Gateway Out  inherit_from_input=off  hdl_port=off  timing_constraint=None  locs_specified=off  LOCs={}  UseAsDAC=off  DACChannel='1'  block_type=gatewayout
  gw_dout : xbsIndex_r4/Gateway Out  inherit_from_input=off  hdl_port=off  timing_constraint=None  locs_specified=off  LOCs={}  UseAsDAC=off  DACChannel='1'  block_type=gatewayout
  gw_vout : xbsIndex_r4/Gateway Out  inherit_from_input=off  hdl_port=off  timing_constraint=None  locs_specified=off  LOCs={}  UseAsDAC=off  DACChannel='1'  block_type=gatewayout
  wires:
  gw_acc_count/1 -> To Workspace3/1
  acc_count/1 -> gw_acc_count/1
  acc_count_pps/1 -> gw_acc_count_pps/1
  gw_acc_end/1 -> To Workspace2/1
  dout/1 -> BitBasher1/1
  gw_acc_count_pps/1 -> To Workspace4/1
  gw_dout/1 -> To Workspace/1
  BitBasher1/1 -> gw_dout/1  "dout"
  vout/1 -> gw_vout/1
  gw_vout/1 -> To Workspace1/1
  acc_end/1 -> gw_acc_end/1

== system x_vacc_test/op_buffer0_orig/edge_detect ==
  in : Inport
  Delay : xbsIndex_r4/Delay  rst=off  infoeditControl=Selection of Reset will increase slice count due to use of real FFs and instead of SRLs  en=off  latency=1  dbl_ovrd=off  reg_retiming=on  block_type=delay
      settings: xl_area=[0, 0, 0, 0, 0, 0, 0]
  Inverter : xbsIndex_r4/Inverter  en=off  latency=0  dbl_ovrd=off  block_type=inv
      settings: xl_area=[0, 0, 0, 0, 0, 0, 0]
  edge_op : xbsIndex_r4/Logical  logical_function=AND  inputs=2  en=off  latency=0  precision=Full  arith_type=Unsigned  n_bits=8  bin_pt=2  align_bp=on  dbl_ovrd=off  block_type=logical
      settings: xl_area=[0, 0, 0, 0, 0, 0, 0]
  out : Outport
  wires:
  Inverter/1 -> edge_op/1
  edge_op/1 -> out/1
  in/1 -> Inverter/1, Delay/1
  Delay/1 -> edge_op/2

== system x_vacc_test/packetizer_x/delayed_pulse ==
  pack_eof : Inport
  In2 : Inport  Port=2
  AddSub1 : xbsIndex_r4/AddSub  mode=Subtraction  use_carryin=off  use_carryout=off  en=off  latency=1  precision=User Defined  arith_type=Unsigned  n_bits=16  bin_pt=0  quantization=Truncate  overflow=Wrap  dbl_ovrd=off  use_behavioral_HDL=off  hw_selection=Fabric  pipelined=off  block_type=addsub
  Constant5 : xbsIndex_r4/Constant  const=3  gui_display_data_type=Fixed-point  arith_type=Unsigned  n_bits=4  bin_pt=0  preci_type=Single  exp_width=8  frac_width=24  explicit_period=on  period=1  equ=P=C  opselect=C  inp2=PCIN>>17  opr=+  inp1=P  carry=CIN  dbl_ovrd=off  block_type=constant
      settings: block_version=11.4
  Convert4 : xbsIndex_r4/Convert  gui_display_data_type=Fixed-point  arith_type=Boolean  n_bits=1  bin_pt=0  float_type=Single  exp_bits=8  fraction_bits=24  quantization=Truncate  overflow=Wrap  en=off  latency=1  dbl_ovrd=off  pipeline=on  block_type=convert
      settings: block_version=11.4
  Count_data3 : xbsIndex_r4/Counter  cnt_type=Free Running  cnt_to=0  operation=Up  start_count=0  cnt_by_val=1  arith_type=Unsigned  n_bits=16  bin_pt=0  load_pin=off  rst=on  en=off  explicit_period=on  period=1  dbl_ovrd=off  use_behavioral_HDL=on  implementation=Fabric  use_rpm=off  block_type=counter
  Count_data4 : xbsIndex_r4/Counter  cnt_type=Free Running  cnt_to=0  operation=Up  start_count=0  cnt_by_val=1  arith_type=Unsigned  n_bits=1  bin_pt=0  load_pin=off  rst=on  en=on  explicit_period=off  period=1  dbl_ovrd=off  use_behavioral_HDL=on  implementation=Fabric  use_rpm=off  block_type=counter
  Inverter2 : xbsIndex_r4/Inverter  en=off  latency=0  dbl_ovrd=off  block_type=inv
      settings: block_version=11.4
  Logical1 : xbsIndex_r4/Logical  logical_function=AND  inputs=2  en=off  latency=0  precision=Full  arith_type=Unsigned  n_bits=16  bin_pt=0  align_bp=on  dbl_ovrd=off  block_type=logical
      settings: block_version=11.4
  Logical6 : xbsIndex_r4/Logical  logical_function=AND  inputs=2  en=off  latency=1  precision=Full  arith_type=Unsigned  n_bits=16  bin_pt=0  align_bp=on  dbl_ovrd=off  block_type=logical
      settings: block_version=11.4
  Relational3 : xbsIndex_r4/Relational  mode=a=b  en=off  latency=1  dbl_ovrd=off  block_type=relational
  delayed_eof : Outport
  wires:
  pack_eof/1 -> Inverter2/1, Count_data3/1, Count_data4/2
  Count_data3/1 -> Relational3/1
  Count_data4/1 -> Convert4/1
  Logical6/1 -> delayed_eof/1, Logical1/1
  Constant5/1 -> AddSub1/2
  AddSub1/1 -> Relational3/2
  In2/1 -> AddSub1/1
  Inverter2/1 -> Logical1/2
  Logical1/1 -> Count_data4/1
  Convert4/1 -> Logical6/1
  Relational3/1 -> Logical6/2

== system x_vacc_test/packetizer_x/edge_detect1 ==
  in : Inport
  Delay : xbsIndex_r4/Delay  rst=off  infoeditControl=Selection of Reset will increase slice count due to use of real FFs and instead of SRLs  en=off  latency=1  dbl_ovrd=off  reg_retiming=on  block_type=delay
      settings: xl_area=[0, 0, 0, 0, 0, 0, 0]
  Inverter : xbsIndex_r4/Inverter  en=off  latency=0  dbl_ovrd=off  block_type=inv
      settings: xl_area=[0, 0, 0, 0, 0, 0, 0]
  edge_op : xbsIndex_r4/Logical  logical_function=NOR  inputs=2  en=off  latency=0  precision=Full  arith_type=Unsigned  n_bits=8  bin_pt=2  align_bp=on  dbl_ovrd=off  block_type=logical
      settings: xl_area=[0, 0, 0, 0, 0, 0, 0]
  out : Outport
  wires:
  Delay/1 -> edge_op/2
  in/1 -> Delay/1, Inverter/1
  edge_op/1 -> out/1
  Inverter/1 -> edge_op/1

== system x_vacc_test/packetizer_x/edge_detect2 ==
  in : Inport
  Delay : xbsIndex_r4/Delay  rst=off  infoeditControl=Selection of Reset will increase slice count due to use of real FFs and instead of SRLs  en=off  latency=1  dbl_ovrd=off  reg_retiming=on  block_type=delay
      settings: xl_area=[0, 0, 0, 0, 0, 0, 0]
  Inverter : xbsIndex_r4/Inverter  en=off  latency=0  dbl_ovrd=off  block_type=inv
      settings: xl_area=[0, 0, 0, 0, 0, 0, 0]
  edge_op : xbsIndex_r4/Logical  logical_function=NOR  inputs=2  en=off  latency=0  precision=Full  arith_type=Unsigned  n_bits=8  bin_pt=2  align_bp=on  dbl_ovrd=off  block_type=logical
      settings: xl_area=[0, 0, 0, 0, 0, 0, 0]
  out : Outport
  wires:
  Inverter/1 -> edge_op/1
  edge_op/1 -> out/1
  in/1 -> Inverter/1, Delay/1
  Delay/1 -> edge_op/2
